simulink model "qrotorBasic"  (R2018b)
settings: Solver=ode15s  StartTime=0.0  StopTime=20  RelTol=1e-3
InitFcn:
  | run('params.m');
values from neighbouring files (symbols this model uses):
  A = [[-hat(Omegad), I];      [O, pinv(J) * (hat(J * Omegad) - hat(Omegad) * J)]]    [attd_simulation.m]    (also in lqr_gains.m = [[-hat(Omegad), I]; params.m = zeros(4,4))
  A_offset = A_offset+[cf_, l_*sin(th_(i))*cf_, -l_*cos(th_(i))*cf_, d_(i)*cm_]'    [params.m]
  F_MAX = tmp_f_max(1)    [params.m]
  F_MIN = tmp_f_min(1)    [params.m]
  g = 9.80665    [params.m]
  invA = pinv(A)    [params.m]
  kd_att = 1.5*[0.12, 0.12, 0.15]'    [params.m]
  kd_pos = 5*[0.5, 0.5, 0.5]'    [params.m]
  ki_pos = 0*[1,1,2]'    [params.m]
  kp_att = 1.8*[0.7, 0.7, 0.25*0.7943]'    [params.m]
  kp_pos = 1*[1,1,5]'    [params.m]
  m = 0.775    [params.m]
  M_MAX = [tmp_mx_max(2); tmp_my_max(3); tmp_mz_max(4)]    [params.m]
  M_MIN = [tmp_mx_min(2); tmp_my_min(3); tmp_mz_min(4)]    [params.m]
  pwm2w = 1166.3755960945295556    (= 1166.37559609)    [params.m]
  pwm2w_offset = -1156.5120044647383111    (= -1156.51200446)    [params.m]
  tau = 0.002    [params.m]
  w2pwm = 0.0008514439377261    (= 0.000851443937726)    [params.m]
  w2pwm_offset = 0.9946035424300900    (= 0.99460354243)    [params.m]
  xInit = [x0 ... (first row only)    [params.m]
libraries: simulink x3, dspindex x2
settings Model: Creator=prasa  LastModifiedBy=kotaru

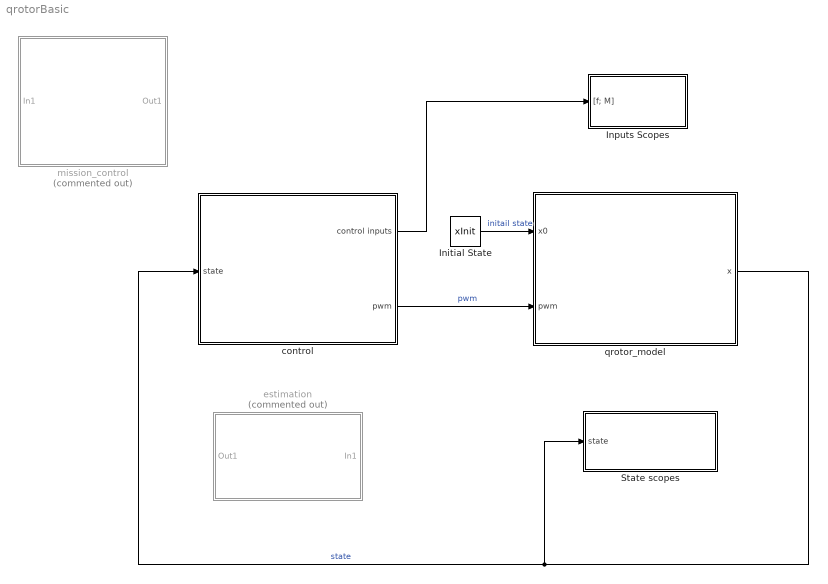
== system qrotorBasic ==
  Initial State : Constant  Value=xInit
  Inputs Scopes : Subsystem
  State scopes : Subsystem
  control : Subsystem
  estimation : Subsystem  Commented=on
      mask Display:
      | ud=get_param(gcb,'UserData');
      | image(ud.z);
  mission_control : Subsystem  Commented=on
      mask Display:
      | ud=get_param(gcb,'UserData');
      | image(ud.y);
  qrotor_model : Subsystem
      mask Display:
      | ud=get_param(gcb,'UserData');
      | image(ud.x);
  wires:
  control/2(pwm) -> qrotor_model/2(pwm)  "pwm"
  qrotor_model/1(x) -> control/1(state), State scopes/1(state)  "state"
  Initial State/1 -> qrotor_model/1(x0)  "initail state"
  control/1(control inputs) -> Inputs Scopes/1("[f; M]")

== system qrotorBasic/Inputs Scopes ==
  note: Control Input Scopes
  "[f; M]" : Inport
  Demux4 : Demux
  Mux : Mux  Inputs=3
  moment scope : Scope
  thrust scope : Scope
  wires:
  Demux4/3 -> Mux/2  "My"
  Mux/1 -> moment scope/1
  "[f; M]"/1 -> Demux4/1
  Demux4/4 -> Mux/3  "Mz"
  Demux4/1 -> thrust scope/1
  Demux4/2 -> Mux/1  "Mx"

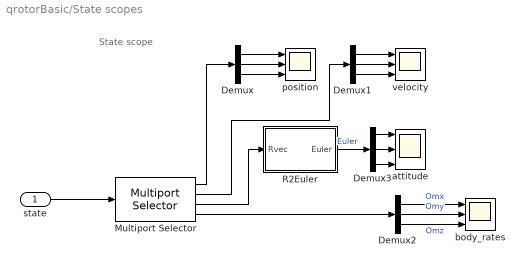
== system qrotorBasic/State scopes ==
  note: State scope
  state : Inport
  Demux{,1..3} (4 alike) : Demux  Outputs=3
  Multiport Selector : dspindex/Multiport Selector  rowsOrCols=Rows  idxCellArray={ [1:3],[4:6],[7:15],[16:18] }  idxErrMode=Clip Index
  R2Euler : MATLAB Function  TreatAsAtomicUnit=on
  "attitude " : Scope  NumInputPorts=3
  body_rates : Scope  NumInputPorts=3
  "position " : Scope  NumInputPorts=3
  velocity : Scope  NumInputPorts=3
  wires:
  Demux/3 -> "position "/3
  Demux/2 -> "position "/2
  Demux/1 -> "position "/1
  Multiport Selector/2 -> Demux1/1
  state/1 -> Multiport Selector/1
  Multiport Selector/1 -> Demux/1
  Demux2/2 -> body_rates/2  "Omy"
  Multiport Selector/3 -> R2Euler/1(Rvec)
  Demux3/1 -> "attitude "/1
  Demux1/2 -> velocity/2
  R2Euler/1(Euler) -> Demux3/1  "Euler"
  Multiport Selector/4 -> Demux2/1
  Demux1/3 -> velocity/3
  Demux2/1 -> body_rates/1  "Omx"
  Demux3/3 -> "attitude "/3
  Demux2/3 -> body_rates/3  "Omz"
  Demux3/2 -> "attitude "/2
  Demux1/1 -> velocity/1

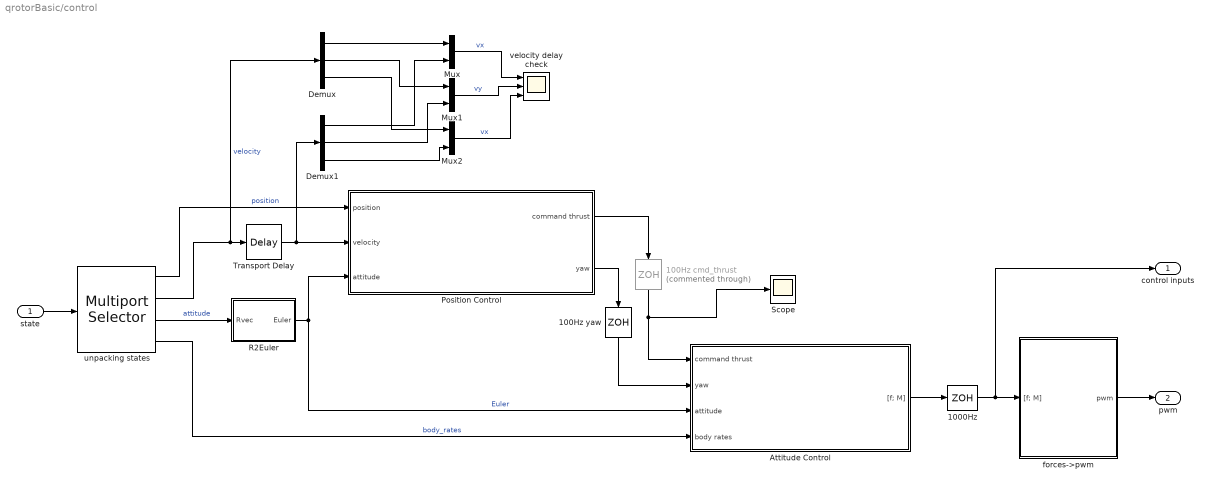
== system qrotorBasic/control ==
  state : Inport
  1000Hz : ZeroOrderHold  SampleTime=0.001
  100Hz cmd_thrust : ZeroOrderHold  Commented=through  SampleTime=0.01
  100Hz yaw : ZeroOrderHold  SampleTime=0.01
  "Attitude Control " : Subsystem
  Demux : Demux  Outputs=3
  Demux1 : Demux  Outputs=3
  Mux : Mux  Inputs=2
  Mux1 : Mux  Inputs=2
  Mux2 : Mux  Inputs=2
  Position Control : Subsystem
  R2Euler : MATLAB Function  TreatAsAtomicUnit=on
  Scope : Scope
  Transport Delay : TransportDelay  DelayTime=0.03
  forces->pwm : Subsystem
  unpacking states : dspindex/Multiport Selector  rowsOrCols=Rows  idxCellArray={ [1:3],[4:6],[7:15],[16:18] }  idxErrMode=Clip Index
  velocity delay check : Scope  NumInputPorts=3
  control inputs : Outport  PortDimensions=4
  pwm : Outport  Port=2  PortDimensions=4
  wires:
  unpacking states/4 -> "Attitude Control "/4(body rates)  "body_rates"
  unpacking states/2 -> Transport Delay/1, Demux/1  "velocity"
  unpacking states/1 -> Position Control/1(position)  "position"
  state/1 -> unpacking states/1
  unpacking states/3 -> R2Euler/1(Rvec)  "attitude"
  "Attitude Control "/1("[f; M]") -> 1000Hz/1
  Position Control/1(command thrust) -> 100Hz cmd_thrust/1
  100Hz cmd_thrust/1 -> Scope/1, "Attitude Control "/1(command thrust)
  Position Control/2(yaw) -> 100Hz yaw/1
  100Hz yaw/1 -> "Attitude Control "/2(yaw)
  R2Euler/1(Euler) -> Position Control/3(attitude), "Attitude Control "/3(attitude)  "Euler"
  forces->pwm/1(pwm) -> pwm/1
  1000Hz/1 -> control inputs/1, forces->pwm/1("[f; M]")
  Transport Delay/1 -> Demux1/1, Position Control/2(velocity)
  Mux/1 -> velocity delay check/1  "vx"
  Mux1/1 -> velocity delay check/2  "vy"
  Mux2/1 -> velocity delay check/3  "vx"
  Demux/1 -> Mux/1
  Demux1/1 -> Mux/2
  Demux/2 -> Mux1/1
  Demux/3 -> Mux2/1
  Demux1/2 -> Mux1/2
  Demux1/3 -> Mux2/2

== system qrotorBasic/estimation ==
  In1 : Inport
  Out1 : Outport
  wires:
  In1/1 -> Out1/1

== system qrotorBasic/mission_control ==
  In1 : Inport
  Out1 : Outport
  wires:
  In1/1 -> Out1/1

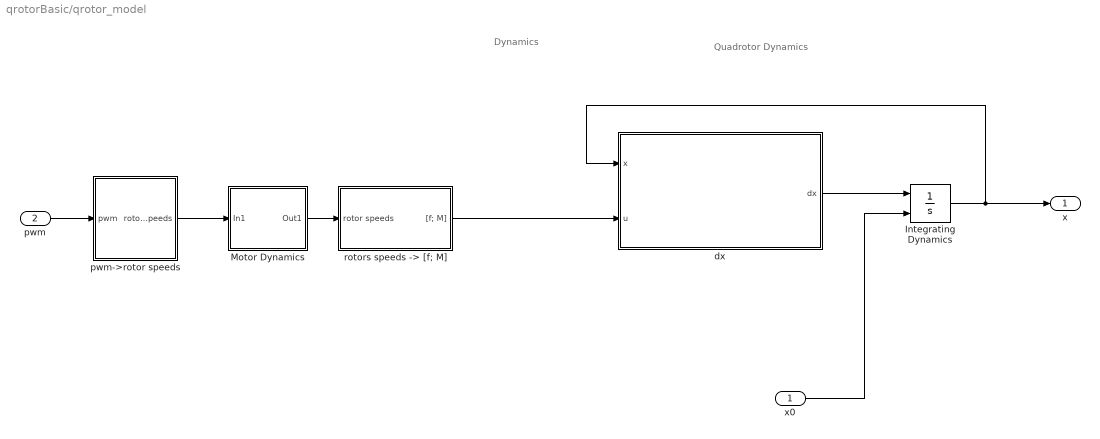
== system qrotorBasic/qrotor_model ==
  note: Quadrotor Dynamics
  note: Dynamics
  x0 : Inport
  pwm : Inport  Port=2  PortDimensions=4
  Integrating Dynamics : Integrator  InitialConditionSource=external  InitialCondition=xInit
  Motor Dynamics : Subsystem
  dx : MATLAB Function  TreatAsAtomicUnit=on
  pwm->rotor speeds : Subsystem
  "rotors speeds -> [f; M]" : Subsystem
  x : Outport
  wires:
  Integrating Dynamics/1 -> dx/1(x), x/1
  dx/1(dx) -> Integrating Dynamics/1
  pwm/1 -> pwm->rotor speeds/1(pwm)
  x0/1 -> Integrating Dynamics/2
  pwm->rotor speeds/1(rotor speeds) -> Motor Dynamics/1(In1)
  Motor Dynamics/1(Out1) -> "rotors speeds -> [f; M]"/1(rotor speeds)
  "rotors speeds -> [f; M]"/1("[f; M]") -> dx/2(u)

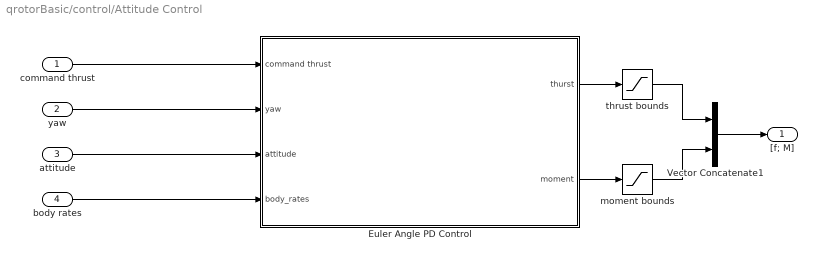
== system qrotorBasic/control/Attitude Control  ==
  command thrust : Inport  PortDimensions=3
  yaw : Inport  Port=2
  attitude : Inport  Port=3  PortDimensions=3
  body rates : Inport  Port=4  PortDimensions=3
  Euler Angle PD Control : Subsystem
  Vector Concatenate1 : Concatenate
  moment bounds : Saturate  UpperLimit=M_MAX  LowerLimit=M_MIN
  thrust bounds : Saturate  UpperLimit=F_MAX  LowerLimit=F_MIN
  "[f; M]" : Outport  PortDimensions=4
  wires:
  Vector Concatenate1/1 -> "[f; M]"/1
  command thrust/1 -> Euler Angle PD Control/1(command thrust)
  yaw/1 -> Euler Angle PD Control/2(yaw)
  attitude/1 -> Euler Angle PD Control/3(attitude)
  body rates/1 -> Euler Angle PD Control/4(body_rates)
  Euler Angle PD Control/1(thurst) -> thrust bounds/1
  Euler Angle PD Control/2(moment) -> moment bounds/1
  thrust bounds/1 -> Vector Concatenate1/1
  moment bounds/1 -> Vector Concatenate1/2

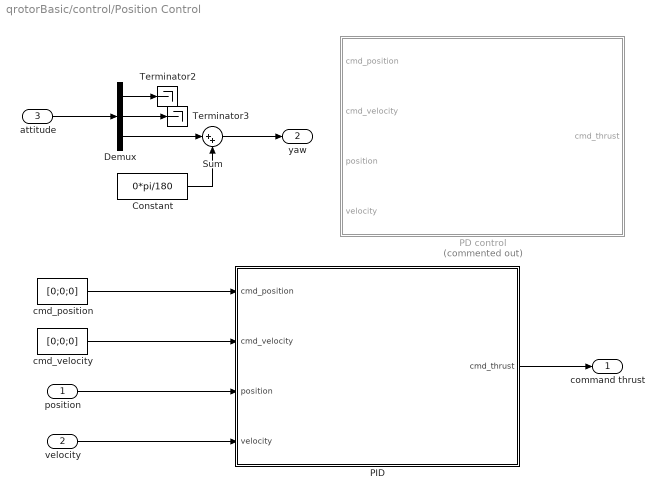
== system qrotorBasic/control/Position Control ==
  position : Inport
  velocity : Inport  Port=2
  attitude : Inport  Port=3
  Constant : Constant  Value=0*pi/180
  Demux : Demux  Outputs=3
  PD control : Subsystem  Commented=on
  PID : Subsystem
  Sum : Sum  Inputs=|++
  Terminator2 : Terminator
  Terminator3 : Terminator
  cmd_position : Constant  Value=[0;0;0]
  cmd_velocity : Constant  Value=[0;0;0]
  command thrust : Outport
  yaw : Outport  Port=2
  wires:
  cmd_position/1 -> PID/1(cmd_position)
  cmd_velocity/1 -> PID/2(cmd_velocity)
  position/1 -> PID/3(position)
  velocity/1 -> PID/4(velocity)
  PID/1(cmd_thrust) -> command thrust/1
  Demux/2 -> Terminator3/1
  Demux/3 -> Sum/1
  attitude/1 -> Demux/1
  Demux/1 -> Terminator2/1
  Sum/1 -> yaw/1
  Constant/1 -> Sum/2

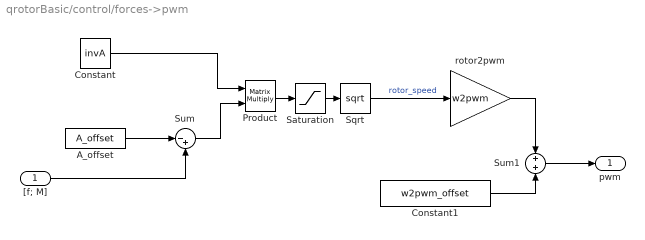
== system qrotorBasic/control/forces->pwm ==
  "[f; M]" : Inport
  A_offset : Constant  Value=A_offset
  Constant : Constant  Value=invA
  Constant1 : Constant  Value=w2pwm_offset
  Product : Product  Multiplication=Matrix(*)
  Saturation : Saturate  UpperLimit=inf  LowerLimit=0
  Sqrt : Sqrt  OutputSignalType=real  SaturateOnIntegerOverflow=on
  Sum : Sum  Inputs=|-+
  Sum1 : Sum
  rotor2pwm : Gain  Gain=w2pwm
  pwm : Outport
  wires:
  rotor2pwm/1 -> Sum1/1
  "[f; M]"/1 -> Sum/2
  A_offset/1 -> Sum/1
  Sum/1 -> Product/2
  Constant/1 -> Product/1
  Product/1 -> Saturation/1
  Sqrt/1 -> rotor2pwm/1  "rotor_speed"
  Sum1/1 -> pwm/1
  Constant1/1 -> Sum1/2
  Saturation/1 -> Sqrt/1

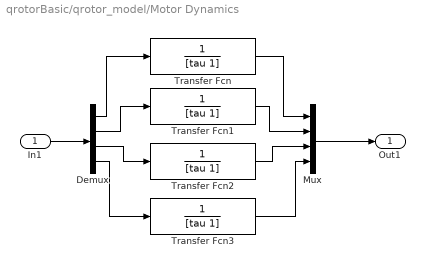
== system qrotorBasic/qrotor_model/Motor Dynamics ==
  In1 : Inport
  Demux : Demux
  Mux : Mux
  Transfer Fcn{,1..3} (4 alike) : TransferFcn  Denominator=[tau 1]
  Out1 : Outport
  wires:
  In1/1 -> Demux/1
  Mux/1 -> Out1/1
  Demux/2 -> Transfer Fcn1/1
  Demux/1 -> Transfer Fcn/1
  Demux/3 -> Transfer Fcn2/1
  Demux/4 -> Transfer Fcn3/1
  Transfer Fcn/1 -> Mux/1
  Transfer Fcn1/1 -> Mux/2
  Transfer Fcn2/1 -> Mux/3
  Transfer Fcn3/1 -> Mux/4

== system qrotorBasic/qrotor_model/pwm->rotor speeds ==
  pwm : Inport
  Constant : Constant  Value=pwm2w_offset
  Gain : Gain  Gain=pwm2w
  Sum : Sum
  rotor speeds : Outport
  wires:
  Gain/1 -> Sum/2
  pwm/1 -> Gain/1
  Constant/1 -> Sum/1
  Sum/1 -> rotor speeds/1

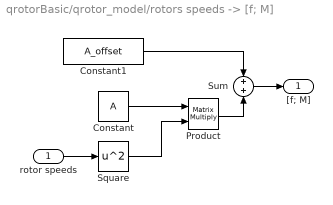
== system qrotorBasic/qrotor_model/rotors speeds -> [f; M] ==
  rotor speeds : Inport
  Constant : Constant  Value=A
  Constant1 : Constant  Value=A_offset
  Product : Product  Multiplication=Matrix(*)
  Square : Math  Operator=square  OutputSignalType=real  SaturateOnIntegerOverflow=on
  Sum : Sum
  "[f; M]" : Outport
  wires:
  rotor speeds/1 -> Square/1
  Sum/1 -> "[f; M]"/1
  Square/1 -> Product/2
  Constant/1 -> Product/1
  Product/1 -> Sum/2
  Constant1/1 -> Sum/1

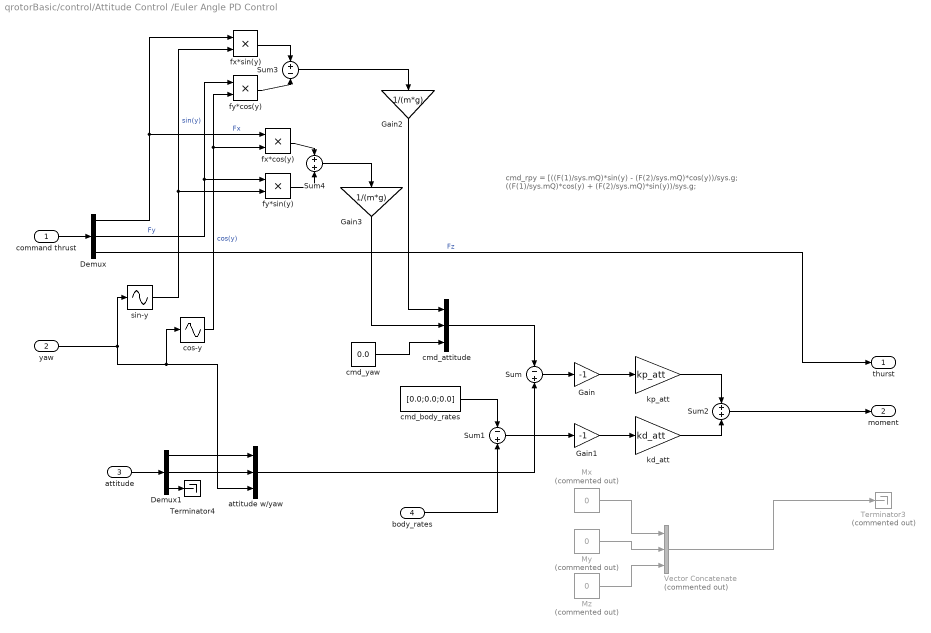
== system qrotorBasic/control/Attitude Control /Euler Angle PD Control ==
  note: cmd_rpy = [((F(1)/sys.mQ)*sin(y) - (F(2)/sys.mQ)*cos(y))/sys.g;
      |                    ((F(1)/sys.mQ)*cos(y) + (F(2)/sys.mQ)*sin(y))/sys.g;
  command thrust : Inport
  yaw : Inport  Port=2
  attitude : Inport  Port=3
  body_rates : Inport  Port=4
  Demux : Demux  Outputs=3
  Demux1 : Demux  Outputs=3
  Gain : Gain  Gain=-1
  Gain1 : Gain  Gain=-1
  Gain2 : Gain  Gain=1/(m*g)
  Gain3 : Gain  Gain=1/(m*g)
  "Mx " : Constant  Commented=on  Value=0
  My : Constant  Commented=on  Value=0
  Mz : Constant  Commented=on  Value=0
  Sum : Sum  Inputs=-+
  Sum1 : Sum  Inputs=-+
  Sum2 : Sum
  Sum3 : Sum  Inputs=+-
  Sum4 : Sum
  Terminator3 : Terminator  Commented=on
  Terminator4 : Terminator
  Vector Concatenate : Concatenate  Commented=on  NumInputs=3
  attitude w/yaw : Mux  Inputs=3
  "cmd_attitude " : Mux  Inputs=3
  cmd_body_rates : Constant  Value=[0.0;0.0;0.0]
  cmd_yaw : Constant  Value=0.0
  cos-y : Sin  TimeSource=Use external signal  Phase=pi/2  SampleTime=0
  fx*cos(y) : Product
  fx*sin(y) : Product
  fy*cos(y) : Product
  fy*sin(y) : Product
  kd_att : Gain  Gain=kd_att
  kp_att : Gain  Gain=kp_att
  sin-y : Sin  TimeSource=Use external signal  SampleTime=0
  thurst : Outport
  moment : Outport  Port=2
  wires:
  Demux/2 -> fy*sin(y)/1, fy*cos(y)/1  "Fy"
  command thrust/1 -> Demux/1
  attitude/1 -> Demux1/1
  yaw/1 -> attitude w/yaw/3, cos-y/1, sin-y/1
  body_rates/1 -> Sum1/2
  Demux/1 -> fx*cos(y)/1, fx*sin(y)/1  "Fx"
  "cmd_attitude "/1 -> Sum/1
  cmd_body_rates/1 -> Sum1/1
  Sum/1 -> Gain/1
  Sum1/1 -> Gain1/1
  Mz/1 -> Vector Concatenate/3
  Vector Concatenate/1 -> Terminator3/1
  My/1 -> Vector Concatenate/2
  "Mx "/1 -> Vector Concatenate/1
  kp_att/1 -> Sum2/1
  kd_att/1 -> Sum2/2
  Sum2/1 -> moment/1
  Demux/3 -> thurst/1  "Fz"
  Gain1/1 -> kd_att/1
  Gain/1 -> kp_att/1
  cmd_yaw/1 -> "cmd_attitude "/3
  Demux1/3 -> Terminator4/1
  Demux1/2 -> attitude w/yaw/2
  Demux1/1 -> attitude w/yaw/1
  attitude w/yaw/1 -> Sum/2
  sin-y/1 -> fy*sin(y)/2, fx*sin(y)/2  "sin(y)"
  cos-y/1 -> fx*cos(y)/2, fy*cos(y)/2  "cos(y)"
  fy*cos(y)/1 -> Sum3/2
  fx*sin(y)/1 -> Sum3/1
  Sum3/1 -> Gain2/1
  Gain2/1 -> "cmd_attitude "/1
  Gain3/1 -> "cmd_attitude "/2
  Sum4/1 -> Gain3/1
  fy*sin(y)/1 -> Sum4/2
  fx*cos(y)/1 -> Sum4/1

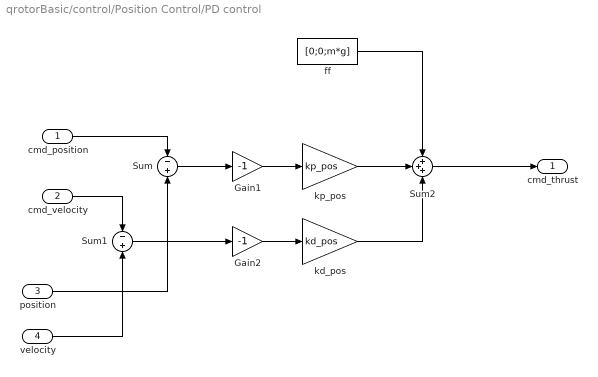
== system qrotorBasic/control/Position Control/PD control ==
  cmd_position : Inport
  cmd_velocity : Inport  Port=2
  position : Inport  Port=3
  velocity : Inport  Port=4
  Gain1 : Gain  Gain=-1
  Gain2 : Gain  Gain=-1
  Sum : Sum  Inputs=-+
  Sum1 : Sum  Inputs=-+
  Sum2 : Sum  Inputs=+++
  ff : Constant  Value=[0;0;m*g]
  kd_pos : Gain  Gain=kd_pos
  kp_pos : Gain  Gain=kp_pos
  cmd_thrust : Outport
  wires:
  ff/1 -> Sum2/1
  Gain1/1 -> kp_pos/1
  kp_pos/1 -> Sum2/2
  cmd_position/1 -> Sum/1
  velocity/1 -> Sum1/2
  position/1 -> Sum/2
  Sum2/1 -> cmd_thrust/1
  cmd_velocity/1 -> Sum1/1
  Sum/1 -> Gain1/1
  kd_pos/1 -> Sum2/3
  Gain2/1 -> kd_pos/1
  Sum1/1 -> Gain2/1

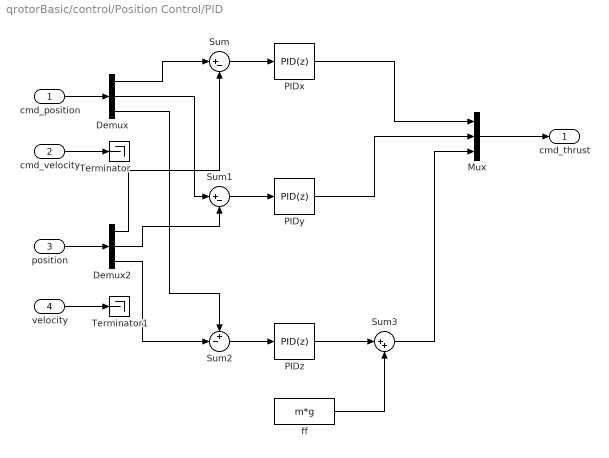
== system qrotorBasic/control/Position Control/PID ==
  cmd_position : Inport
  cmd_velocity : Inport  Port=2
  position : Inport  Port=3
  velocity : Inport  Port=4
  Demux : Demux  Outputs=3
  Demux2 : Demux  Outputs=3
  Mux : Mux  Inputs=3
  PIDx : simulink/Continuous/PID Controller  Controller=PID  Form=Parallel  TimeDomain=Discrete-time  SampleTime=0.1  IntegratorMethod=Forward Euler  FilterMethod=Forward Euler  ControllerParametersSource=internal  P=kp_pos(1)  I=ki_pos(1)  D=kd_pos(1)  UseFilter=off  N=100  TunerSelectOption=Transfer Function Based (PID Tuner App)  InitialConditionSource=internal  InitialConditionForIntegrator=0  InitialConditionForFilter=0  DifferentiatorICPrevScaledInput=0  ExternalReset=none  TrackingMode=off  Kt=1  LimitOutput=off  UpperSaturationLimit=inf  LowerSaturationLimit=-inf  LinearizeAsGain=off  AntiWindupMode=none  Kb=1  POutMin=[]  POutMax=[]  IOutMin=[]  IOutMax=[]  DOutMin=[]  DOutMax=[]  NOutMin=[]  NOutMax=[]  SumOutMin=[]  SumOutMax=[]  SaturationOutMin=[]  SaturationOutMax=[]  PParamMin=[]  PParamMax=[]  IParamMin=[]  IParamMax=[]  DParamMin=[]  DParamMax=[]  NParamMin=[]  NParamMax=[]  KbParamMin=[]  KbParamMax=[]  KtParamMin=[]  KtParamMax=[]  KbOutMin=[]  KbOutMax=[]  KtOutMin=[]  KtOutMax=[]  IntegratorOutMin=[]  IntegratorOutMax=[]  FilterOutMin=[]  FilterOutMax=[]  SumI1OutMin=[]  SumI1OutMax=[]  SumI2OutMin=[]  SumI2OutMax=[]  SumI3OutMin=[]  SumI3OutMax=[]  SumI4OutMin=[]  SumI4OutMax=[]  SumDOutMin=[]  SumDOutMax=[]  DifferentiatorOutMin=[]  DifferentiatorOutMax=[]  FilterDiffOutCoefMin=[]  FilterDiffOutCoefMax=[]  ReciprocalOutMin=[]  ReciprocalOutMax=[]  SumDenOutMin=[]  SumDenOutMax=[]  SumNumOutMin=[]  SumNumOutMax=[]  DivideOutMin=[]  DivideOutMax=[]  IntegratorContinuousStateAttributes=''  IntegratorStateMustResolveToSignalObject=off  IntegratorRTWStateStorageClass=Auto  FilterContinuousStateAttributes=''  FilterStateMustResolveToSignalObject=off  FilterRTWStateStorageClass=Auto  ParallelPVariant=InternalParameters  IdealPVariant=Disabled  IVariant=InternalParameters  DVariant=InternalParameters  IntegratorVariant=Discrete  SatVariant=Disabled  AWVariant=Passthrough  PCopyVariant=Disabled  TRVariant=Disabled  FdbkBlocksVariant=Forward  IdealPFdbkVariant=Disabled  SatFdbkVariant=Disabled  DerivativeFilterVariant=Differentiator  NVariant=Passthrough  NCopyVariant=Disabled_SignalSpec  FilterICVariant=InternalICsDifferentiator  IntegratorICVariant=InternalICs  ExternalResetVariant=Disabled  TRSumVariant=Passthrough  SumFdbkVariant=Disabled  SumVariant=Sum_PID
  PIDy : simulink/Continuous/PID Controller  Controller=PID  Form=Parallel  TimeDomain=Discrete-time  SampleTime=0.1  IntegratorMethod=Forward Euler  FilterMethod=Forward Euler  ControllerParametersSource=internal  P=kp_pos(2)  I=ki_pos(2)  D=kd_pos(2)  UseFilter=off  N=100  TunerSelectOption=Transfer Function Based (PID Tuner App)  InitialConditionSource=internal  InitialConditionForIntegrator=0  InitialConditionForFilter=0  DifferentiatorICPrevScaledInput=0  ExternalReset=none  TrackingMode=off  Kt=1  LimitOutput=off  UpperSaturationLimit=inf  LowerSaturationLimit=-inf  LinearizeAsGain=off  AntiWindupMode=none  Kb=1  POutMin=[]  POutMax=[]  IOutMin=[]  IOutMax=[]  DOutMin=[]  DOutMax=[]  NOutMin=[]  NOutMax=[]  SumOutMin=[]  SumOutMax=[]  SaturationOutMin=[]  SaturationOutMax=[]  PParamMin=[]  PParamMax=[]  IParamMin=[]  IParamMax=[]  DParamMin=[]  DParamMax=[]  NParamMin=[]  NParamMax=[]  KbParamMin=[]  KbParamMax=[]  KtParamMin=[]  KtParamMax=[]  KbOutMin=[]  KbOutMax=[]  KtOutMin=[]  KtOutMax=[]  IntegratorOutMin=[]  IntegratorOutMax=[]  FilterOutMin=[]  FilterOutMax=[]  SumI1OutMin=[]  SumI1OutMax=[]  SumI2OutMin=[]  SumI2OutMax=[]  SumI3OutMin=[]  SumI3OutMax=[]  SumI4OutMin=[]  SumI4OutMax=[]  SumDOutMin=[]  SumDOutMax=[]  DifferentiatorOutMin=[]  DifferentiatorOutMax=[]  FilterDiffOutCoefMin=[]  FilterDiffOutCoefMax=[]  ReciprocalOutMin=[]  ReciprocalOutMax=[]  SumDenOutMin=[]  SumDenOutMax=[]  SumNumOutMin=[]  SumNumOutMax=[]  DivideOutMin=[]  DivideOutMax=[]  IntegratorContinuousStateAttributes=''  IntegratorStateMustResolveToSignalObject=off  IntegratorRTWStateStorageClass=Auto  FilterContinuousStateAttributes=''  FilterStateMustResolveToSignalObject=off  FilterRTWStateStorageClass=Auto  ParallelPVariant=InternalParameters  IdealPVariant=Disabled  IVariant=InternalParameters  DVariant=InternalParameters  IntegratorVariant=Discrete  SatVariant=Disabled  AWVariant=Passthrough  PCopyVariant=Disabled  TRVariant=Disabled  FdbkBlocksVariant=Forward  IdealPFdbkVariant=Disabled  SatFdbkVariant=Disabled  DerivativeFilterVariant=Differentiator  NVariant=Passthrough  NCopyVariant=Disabled_SignalSpec  FilterICVariant=InternalICsDifferentiator  IntegratorICVariant=InternalICs  ExternalResetVariant=Disabled  TRSumVariant=Passthrough  SumFdbkVariant=Disabled  SumVariant=Sum_PID
  PIDz : simulink/Continuous/PID Controller  Controller=PID  Form=Parallel  TimeDomain=Discrete-time  SampleTime=0.1  IntegratorMethod=Forward Euler  FilterMethod=Forward Euler  ControllerParametersSource=internal  P=kp_pos(3)  I=ki_pos(3)  D=kd_pos(3)  UseFilter=off  N=100  TunerSelectOption=Transfer Function Based (PID Tuner App)  InitialConditionSource=internal  InitialConditionForIntegrator=0  InitialConditionForFilter=0  DifferentiatorICPrevScaledInput=0  ExternalReset=none  TrackingMode=off  Kt=1  LimitOutput=off  UpperSaturationLimit=inf  LowerSaturationLimit=-inf  LinearizeAsGain=off  AntiWindupMode=none  Kb=1  POutMin=[]  POutMax=[]  IOutMin=[]  IOutMax=[]  DOutMin=[]  DOutMax=[]  NOutMin=[]  NOutMax=[]  SumOutMin=[]  SumOutMax=[]  SaturationOutMin=[]  SaturationOutMax=[]  PParamMin=[]  PParamMax=[]  IParamMin=[]  IParamMax=[]  DParamMin=[]  DParamMax=[]  NParamMin=[]  NParamMax=[]  KbParamMin=[]  KbParamMax=[]  KtParamMin=[]  KtParamMax=[]  KbOutMin=[]  KbOutMax=[]  KtOutMin=[]  KtOutMax=[]  IntegratorOutMin=[]  IntegratorOutMax=[]  FilterOutMin=[]  FilterOutMax=[]  SumI1OutMin=[]  SumI1OutMax=[]  SumI2OutMin=[]  SumI2OutMax=[]  SumI3OutMin=[]  SumI3OutMax=[]  SumI4OutMin=[]  SumI4OutMax=[]  SumDOutMin=[]  SumDOutMax=[]  DifferentiatorOutMin=[]  DifferentiatorOutMax=[]  FilterDiffOutCoefMin=[]  FilterDiffOutCoefMax=[]  ReciprocalOutMin=[]  ReciprocalOutMax=[]  SumDenOutMin=[]  SumDenOutMax=[]  SumNumOutMin=[]  SumNumOutMax=[]  DivideOutMin=[]  DivideOutMax=[]  IntegratorContinuousStateAttributes=''  IntegratorStateMustResolveToSignalObject=off  IntegratorRTWStateStorageClass=Auto  FilterContinuousStateAttributes=''  FilterStateMustResolveToSignalObject=off  FilterRTWStateStorageClass=Auto  ParallelPVariant=InternalParameters  IdealPVariant=Disabled  IVariant=InternalParameters  DVariant=InternalParameters  IntegratorVariant=Discrete  SatVariant=Disabled  AWVariant=Passthrough  PCopyVariant=Disabled  TRVariant=Disabled  FdbkBlocksVariant=Forward  IdealPFdbkVariant=Disabled  SatFdbkVariant=Disabled  DerivativeFilterVariant=Differentiator  NVariant=Passthrough  NCopyVariant=Disabled_SignalSpec  FilterICVariant=InternalICsDifferentiator  IntegratorICVariant=InternalICs  ExternalResetVariant=Disabled  TRSumVariant=Passthrough  SumFdbkVariant=Disabled  SumVariant=Sum_PID
  Sum : Sum  Inputs=|+-
  Sum1 : Sum  Inputs=|+-
  Sum2 : Sum  Inputs=+-|
  Sum3 : Sum  Inputs=|++
  Terminator : Terminator
  Terminator1 : Terminator
  ff : Constant  Value=m*g
  cmd_thrust : Outport
  wires:
  ff/1 -> Sum3/2
  velocity/1 -> Terminator1/1
  position/1 -> Demux2/1
  cmd_velocity/1 -> Terminator/1
  cmd_position/1 -> Demux/1
  Sum/1 -> PIDx/1
  Sum2/1 -> PIDz/1
  Sum1/1 -> PIDy/1
  Demux2/3 -> Sum2/2
  Demux/3 -> Sum2/1
  Demux2/2 -> Sum1/2
  Demux2/1 -> Sum/2
  Demux/2 -> Sum1/1
  Demux/1 -> Sum/1
  PIDz/1 -> Sum3/1
  Mux/1 -> cmd_thrust/1
  Sum3/1 -> Mux/3
  PIDy/1 -> Mux/2
  PIDx/1 -> Mux/1

== matlab function qrotorBasic/control/R2Euler ==
  data: Rvec input; Euler output
function Euler = R2Euler(Rvec)

R = reshape(Rvec,3,3)';
phi = asin(R(2,3));
psi = atan2(-R(2,1)/cos(phi),R(2,2)/cos(phi));
theta = atan2(-R(1,3)/cos(phi),R(3,3)/cos(phi));

Euler = [phi; theta; psi];

== matlab function qrotorBasic/qrotor_model/dx ==
  data: x input; dx output; u input
function dx = qrotorDyn(x, u)

g = 9.80665;
m = 0.85;
J = [0.005315307431627, 0.000005567447099, 0.000005445855427;
0.000005567447099, 0.004949258422243, 0.000020951458431;
0.000005445855427, 0.000020951458431, 0.009806225007686;];

invJ = [188.1362   -0.2112   -0.1040;
   -0.2112  202.0525   -0.4316;
   -0.1040   -0.4316  101.9770];

e1 = [1; 0; 0];
e2 = [0; 1; 0];
e3 = [0; 0; 1];

xQ = x(1:3,1);
vQ = x(4:6,1);
R  = reshape(x(7:15),3,3);
Om = x(16:18,1);

dOm = invJ*[u(2:4)-cross2(Om,J*Om)];

dx = [vQ; -g*e3+(u(1)*R*e3/m); reshape(R*hat(Om),9,1); dOm];

%%
% Om = x(10:12);
% 
% phi = rpy(1);
% theta = rpy(2);
% psi = rpy(3);
% 
% R = [ cos(psi)*cos(theta) - sin(phi)*sin(psi)*sin(theta),... 
%     cos(theta)*sin(psi) + cos(psi)*sin(phi)*sin(theta), -cos(phi)*sin(theta);...
%     -cos(phi)*sin(psi),...
%     cos(phi)*cos(psi),             sin(phi);...
%     cos(psi)*sin(theta) + cos(theta)*sin(phi)*sin(psi),...
%     sin(psi)*sin(theta) - cos(psi)*cos(theta)*sin(phi),...
%     cos(phi)*cos(theta)]';
% 
% dv = -g*e3 + u(1)*R*e3;
% drpy = Omega2eulerratesMat_ZXY(rpy)*Om;
% dOm = invJ(u(2:4) - cross(Om,J*Om));

% dx = [v; dv; drpy; dOm];

end

% function invA = Omega2eulerratesMat_ZXY(in1)
% %OMEGA2EULERRATESMAT_ZXY
% %    INVA = OMEGA2EULERRATESMAT_ZXY(IN1)
% 
% %    This function was generated by the Symbolic Math Toolbox version 8.2.
% %    27-Sep-2018 17:22:21
% 
% p = in1(2,:);
% r = in1(1,:);
% t2 = sin(p);
% t3 = cos(p);
% t4 = cos(r);
% t5 = 1.0./t4;
% t6 = sin(r);
% invA = reshape([t3,t2.*t5.*t6,-t2.*t5,0.0,1.0,0.0,t2,-t3.*t5.*t6,t3.*t5],[3,3]);

% end

== matlab function qrotorBasic/State scopes/R2Euler ==
  data: Rvec input; Euler output
function Euler = R2Euler(Rvec)

R = reshape(Rvec,3,3)';
phi = asin(R(2,3));
psi = atan2(-R(2,1)/cos(phi),R(2,2)/cos(phi));
theta = atan2(-R(1,3)/cos(phi),R(3,3)/cos(phi));

Euler = [phi; theta; psi];
